simulink model "rfi_blk_v6_casper"  (Simulink 7.1)
settings: Solver=ode45  StartTime=0.0  StopTime=10.0  RelTol=1e-3
undefined symbols (not defined in the file): a, b
libraries: xbsIndex_r4 x13084, xps_library x4
(part 3 of 51)

== system rfi_blk_v6_casper/rfi_blk/mad_comp/Median1/Subsystem11 ==
  data_in : Inport  OutDataType=sfix(16)  OutScaling=2^0
  med_loc : Inport  Port=2  OutDataType=sfix(16)  OutScaling=2^0
  acc_reset : Inport  Port=3  OutDataType=sfix(16)  OutScaling=2^0
  " System Generator" : xbsIndex_r4/ System Generator  Tag=genX  SystemSampleTime=-1  xilinxfamily=Virtex4  part=xc4vsx35  speed=-10  package=ff668  synthesis_tool=XST  clock_wrapper=Clock Enables  directory=./netlist  testbench=off  simulink_period=1  sysclk_period=100  dcm_input_clock_period=100  incr_netlist=off  trim_vbits=Everywhere in SubSystem  dbl_ovrd=According to Block Masks  core_generation=According to Block Masks  run_coregen=off  deprecated_control=off  eval_field=0  block_type=sysgen
      settings: block_version=10.1.3
  Constant10 : xbsIndex_r4/Constant  arith_type=Boolean  const=1  n_bits=1  bin_pt=0  explicit_period=on  period=1  equ=P=C  opselect=C  inp2=PCIN>>17  opr=+  inp1=P  carry=CIN  dbl_ovrd=off  block_type=constant
  Constant11 : xbsIndex_r4/Constant  arith_type=Boolean  const=0  n_bits=1  bin_pt=0  explicit_period=on  period=1  equ=P=C  opselect=C  inp2=PCIN>>17  opr=+  inp1=P  carry=CIN  dbl_ovrd=off  block_type=constant
  Constant12 : xbsIndex_r4/Constant  arith_type=Boolean  const=1  n_bits=1  bin_pt=0  explicit_period=on  period=1  equ=P=C  opselect=C  inp2=PCIN>>17  opr=+  inp1=P  carry=CIN  dbl_ovrd=off  block_type=constant
  Constant2 : xbsIndex_r4/Constant  arith_type=Unsigned  const=0  n_bits=8  bin_pt=0  explicit_period=on  period=1  equ=P=C  opselect=C  inp2=PCIN>>17  opr=+  inp1=P  carry=CIN  dbl_ovrd=off  block_type=constant
  Constant3 : xbsIndex_r4/Constant  arith_type=Unsigned  const=0  n_bits=8  bin_pt=0  explicit_period=on  period=1  equ=P=C  opselect=C  inp2=PCIN>>17  opr=+  inp1=P  carry=CIN  dbl_ovrd=off  block_type=constant
  Constant9 : xbsIndex_r4/Constant  arith_type=Boolean  const=0  n_bits=1  bin_pt=0  explicit_period=on  period=1  equ=P=C  opselect=C  inp2=PCIN>>17  opr=+  inp1=P  carry=CIN  dbl_ovrd=off  block_type=constant
  Inverter : xbsIndex_r4/Inverter  en=off  latency=0  dbl_ovrd=off  block_type=inv
  Logical : xbsIndex_r4/Logical  logical_function=AND  inputs=2  en=off  latency=0  precision=Full  arith_type=Unsigned  n_bits=16  bin_pt=0  align_bp=on  dbl_ovrd=off  block_type=logical
  Logical1 : xbsIndex_r4/Logical  logical_function=OR  inputs=2  en=off  latency=1  precision=User Defined  arith_type=Unsigned  n_bits=1  bin_pt=0  align_bp=on  dbl_ovrd=off  block_type=logical
  Logical2 : xbsIndex_r4/Logical  logical_function=OR  inputs=2  en=off  latency=1  precision=User Defined  arith_type="Signed  (2's comp)"  n_bits=8  bin_pt=0  align_bp=on  dbl_ovrd=off  block_type=logical
  Median_Accumulator1 : Subsystem
  Median_Accumulator2 : Subsystem
  Median_Location1 : Subsystem
  Median_Location2 : Subsystem
  Mux : xbsIndex_r4/Mux  inputs=2  en=off  latency=0  precision=Full  arith_type=Unsigned  n_bits=16  bin_pt=14  quantization=Truncate  overflow=Wrap  dbl_ovrd=off  block_type=mux
  Mux1 : xbsIndex_r4/Mux  inputs=2  en=off  latency=0  precision=Full  arith_type="Signed  (2's comp)"  n_bits=8  bin_pt=0  quantization=Truncate  overflow=Wrap  dbl_ovrd=off  block_type=mux
  Mux2 : xbsIndex_r4/Mux  inputs=2  en=off  latency=0  precision=User Defined  arith_type="Signed  (2's comp)"  n_bits=8  bin_pt=0  quantization=Truncate  overflow=Wrap  dbl_ovrd=off  block_type=mux
  Mux3 : xbsIndex_r4/Mux  inputs=2  en=off  latency=0  precision=User Defined  arith_type="Signed  (2's comp)"  n_bits=8  bin_pt=0  quantization=Truncate  overflow=Wrap  dbl_ovrd=off  block_type=mux
  Scope{1..5} (5 alike) : Scope  NumInputPorts=2
      Scope1 settings: YMin=-5~-5  YMax=5~5
      Scope2 settings: YMin=-5~-5  YMax=5~5
      Scope3 settings: YMin=-5~-5  YMax=5~5  SaveName=ScopeData5
      Scope4 settings: YMin=-5~-5  YMax=5~5  SaveName=ScopeData7
      Scope5 settings: YMin=-5~-5  YMax=5~5  SaveName=ScopeData15
  priority_out1 : Outport  OutDataType=sfix(16)  OutScaling=2^0
  med_calc1 : Outport  Port=2  OutDataType=sfix(16)  OutScaling=2^0
  inc_out : Outport  Port=3
  wires:
  data_in/1 -> Median_Accumulator1/1(data_in), Median_Accumulator2/1(data_in)
  Median_Accumulator1/1(Out1) -> Median_Location1/1(In1), Scope5/1
  Median_Accumulator1/2(Out2) -> Median_Location1/2(In2)
  Median_Accumulator1/3(Out3) -> Median_Location1/3(In3)
  Median_Accumulator1/4(Out4) -> Median_Location1/4(In4)
  Median_Accumulator1/5(Out5) -> Median_Location1/5(In5), Scope5/2
  Median_Accumulator1/6(Out6) -> Median_Location1/6(In6)
  Median_Accumulator1/7(Out7) -> Median_Location1/7(In7)
  Median_Accumulator1/8(Out8) -> Median_Location1/8(In8)
  Median_Location1/1(Out1) -> Scope1/1, Mux2/3
  med_loc/1 -> Median_Location1/9(median_loc), Median_Location2/9(median_loc)
  Median_Accumulator2/8(Out8) -> Median_Location2/8(In8)
  Median_Accumulator2/7(Out7) -> Median_Location2/7(In7)
  Median_Accumulator2/6(Out6) -> Median_Location2/6(In6)
  Median_Accumulator2/5(Out5) -> Median_Location2/5(In5)
  Median_Accumulator2/4(Out4) -> Median_Location2/4(In4)
  Median_Accumulator2/3(Out3) -> Median_Location2/3(In3)
  Median_Accumulator2/2(Out2) -> Median_Location2/2(In2)
  Median_Accumulator2/1(Out1) -> Median_Location2/1(In1)
  Constant9/1 -> Mux/2
  Constant10/1 -> Mux/3
  Mux/1 -> Logical1/1, Mux2/1
  Inverter/1 -> Logical/1
  Logical/1 -> Mux1/1
  Constant11/1 -> Mux1/2
  Mux1/1 -> Logical1/2, Mux3/1
  Median_Location2/1(Out3) -> Mux3/3, Scope2/1, Scope4/2
  Constant12/1 -> Mux1/3
  Mux2/1 -> Logical2/1
  Mux3/1 -> Logical2/2
  Constant2/1 -> Mux2/2
  Constant3/1 -> Mux3/2
  Logical2/1 -> Scope3/2, med_calc1/1
  Logical1/1 -> priority_out1/1, Scope3/1
  Median_Accumulator2/9(inc_signal) -> inc_out/1
  Median_Location1/2(sel1) -> Mux/1, Inverter/1, Scope1/2
  Median_Location2/2(sel2) -> Logical/2, Scope2/2
  acc_reset/1 -> Median_Accumulator1/2(acc_reset), Median_Accumulator2/2(acc_reset)

== system rfi_blk_v6_casper/rfi_blk/mad_comp/Median1/Subsystem12 ==
  data_in : Inport  OutDataType=sfix(16)  OutScaling=2^0
  med_loc : Inport  Port=2  OutDataType=sfix(16)  OutScaling=2^0
  acc_reset : Inport  Port=3  OutDataType=sfix(16)  OutScaling=2^0
  " System Generator" : xbsIndex_r4/ System Generator  Tag=genX  SystemSampleTime=-1  xilinxfamily=Virtex4  part=xc4vsx35  speed=-10  package=ff668  synthesis_tool=XST  clock_wrapper=Clock Enables  directory=./netlist  testbench=off  simulink_period=1  sysclk_period=100  dcm_input_clock_period=100  incr_netlist=off  trim_vbits=Everywhere in SubSystem  dbl_ovrd=According to Block Masks  core_generation=According to Block Masks  run_coregen=off  deprecated_control=off  eval_field=0  block_type=sysgen
      settings: block_version=10.1.3
  Constant10 : xbsIndex_r4/Constant  arith_type=Boolean  const=1  n_bits=1  bin_pt=0  explicit_period=on  period=1  equ=P=C  opselect=C  inp2=PCIN>>17  opr=+  inp1=P  carry=CIN  dbl_ovrd=off  block_type=constant
  Constant11 : xbsIndex_r4/Constant  arith_type=Boolean  const=0  n_bits=1  bin_pt=0  explicit_period=on  period=1  equ=P=C  opselect=C  inp2=PCIN>>17  opr=+  inp1=P  carry=CIN  dbl_ovrd=off  block_type=constant
  Constant12 : xbsIndex_r4/Constant  arith_type=Boolean  const=1  n_bits=1  bin_pt=0  explicit_period=on  period=1  equ=P=C  opselect=C  inp2=PCIN>>17  opr=+  inp1=P  carry=CIN  dbl_ovrd=off  block_type=constant
  Constant2 : xbsIndex_r4/Constant  arith_type=Unsigned  const=0  n_bits=8  bin_pt=0  explicit_period=on  period=1  equ=P=C  opselect=C  inp2=PCIN>>17  opr=+  inp1=P  carry=CIN  dbl_ovrd=off  block_type=constant
  Constant3 : xbsIndex_r4/Constant  arith_type=Unsigned  const=0  n_bits=8  bin_pt=0  explicit_period=on  period=1  equ=P=C  opselect=C  inp2=PCIN>>17  opr=+  inp1=P  carry=CIN  dbl_ovrd=off  block_type=constant
  Constant9 : xbsIndex_r4/Constant  arith_type=Boolean  const=0  n_bits=1  bin_pt=0  explicit_period=on  period=1  equ=P=C  opselect=C  inp2=PCIN>>17  opr=+  inp1=P  carry=CIN  dbl_ovrd=off  block_type=constant
  Inverter : xbsIndex_r4/Inverter  en=off  latency=0  dbl_ovrd=off  block_type=inv
  Logical : xbsIndex_r4/Logical  logical_function=AND  inputs=2  en=off  latency=0  precision=Full  arith_type=Unsigned  n_bits=16  bin_pt=0  align_bp=on  dbl_ovrd=off  block_type=logical
  Logical1 : xbsIndex_r4/Logical  logical_function=OR  inputs=2  en=off  latency=1  precision=User Defined  arith_type=Unsigned  n_bits=1  bin_pt=0  align_bp=on  dbl_ovrd=off  block_type=logical
  Logical2 : xbsIndex_r4/Logical  logical_function=OR  inputs=2  en=off  latency=1  precision=User Defined  arith_type="Signed  (2's comp)"  n_bits=8  bin_pt=0  align_bp=on  dbl_ovrd=off  block_type=logical
  Median_Accumulator1 : Subsystem
  Median_Accumulator2 : Subsystem
  Median_Location1 : Subsystem
  Median_Location2 : Subsystem
  Mux : xbsIndex_r4/Mux  inputs=2  en=off  latency=0  precision=Full  arith_type=Unsigned  n_bits=16  bin_pt=14  quantization=Truncate  overflow=Wrap  dbl_ovrd=off  block_type=mux
  Mux1 : xbsIndex_r4/Mux  inputs=2  en=off  latency=0  precision=Full  arith_type="Signed  (2's comp)"  n_bits=8  bin_pt=0  quantization=Truncate  overflow=Wrap  dbl_ovrd=off  block_type=mux
  Mux2 : xbsIndex_r4/Mux  inputs=2  en=off  latency=0  precision=User Defined  arith_type="Signed  (2's comp)"  n_bits=8  bin_pt=0  quantization=Truncate  overflow=Wrap  dbl_ovrd=off  block_type=mux
  Mux3 : xbsIndex_r4/Mux  inputs=2  en=off  latency=0  precision=User Defined  arith_type="Signed  (2's comp)"  n_bits=8  bin_pt=0  quantization=Truncate  overflow=Wrap  dbl_ovrd=off  block_type=mux
  Scope{1..5} (5 alike) : Scope  NumInputPorts=2
      Scope1 settings: YMin=-5~-5  YMax=5~5
      Scope2 settings: YMin=-5~-5  YMax=5~5
      Scope3 settings: YMin=-5~-5  YMax=5~5  SaveName=ScopeData5
      Scope4 settings: YMin=-5~-5  YMax=5~5  SaveName=ScopeData7
      Scope5 settings: YMin=-5~-5  YMax=5~5  SaveName=ScopeData15
  priority_out1 : Outport  OutDataType=sfix(16)  OutScaling=2^0
  med_calc1 : Outport  Port=2  OutDataType=sfix(16)  OutScaling=2^0
  inc_out : Outport  Port=3
  wires:
  data_in/1 -> Median_Accumulator1/1(data_in), Median_Accumulator2/1(data_in)
  Median_Accumulator1/1(Out1) -> Median_Location1/1(In1), Scope5/1
  Median_Accumulator1/2(Out2) -> Median_Location1/2(In2)
  Median_Accumulator1/3(Out3) -> Median_Location1/3(In3)
  Median_Accumulator1/4(Out4) -> Median_Location1/4(In4)
  Median_Accumulator1/5(Out5) -> Median_Location1/5(In5), Scope5/2
  Median_Accumulator1/6(Out6) -> Median_Location1/6(In6)
  Median_Accumulator1/7(Out7) -> Median_Location1/7(In7)
  Median_Accumulator1/8(Out8) -> Median_Location1/8(In8)
  Median_Location1/1(Out1) -> Scope1/1, Mux2/3
  med_loc/1 -> Median_Location1/9(median_loc), Median_Location2/9(median_loc)
  Median_Accumulator2/8(Out8) -> Median_Location2/8(In8)
  Median_Accumulator2/7(Out7) -> Median_Location2/7(In7)
  Median_Accumulator2/6(Out6) -> Median_Location2/6(In6)
  Median_Accumulator2/5(Out5) -> Median_Location2/5(In5)
  Median_Accumulator2/4(Out4) -> Median_Location2/4(In4)
  Median_Accumulator2/3(Out3) -> Median_Location2/3(In3)
  Median_Accumulator2/2(Out2) -> Median_Location2/2(In2)
  Median_Accumulator2/1(Out1) -> Median_Location2/1(In1)
  Constant9/1 -> Mux/2
  Constant10/1 -> Mux/3
  Mux/1 -> Logical1/1, Mux2/1
  Inverter/1 -> Logical/1
  Logical/1 -> Mux1/1
  Constant11/1 -> Mux1/2
  Mux1/1 -> Logical1/2, Mux3/1
  Median_Location2/1(Out3) -> Mux3/3, Scope2/1, Scope4/2
  Constant12/1 -> Mux1/3
  Mux2/1 -> Logical2/1
  Mux3/1 -> Logical2/2
  Constant2/1 -> Mux2/2
  Constant3/1 -> Mux3/2
  Logical2/1 -> Scope3/2, med_calc1/1
  Logical1/1 -> priority_out1/1, Scope3/1
  Median_Accumulator2/9(inc_signal) -> inc_out/1
  Median_Location1/2(sel1) -> Mux/1, Inverter/1, Scope1/2
  Median_Location2/2(sel2) -> Logical/2, Scope2/2
  acc_reset/1 -> Median_Accumulator1/2(acc_reset), Median_Accumulator2/2(acc_reset)

== system rfi_blk_v6_casper/rfi_blk/mad_comp/Median1/Subsystem13 ==
  data_in : Inport  OutDataType=sfix(16)  OutScaling=2^0
  med_loc : Inport  Port=2  OutDataType=sfix(16)  OutScaling=2^0
  acc_reset : Inport  Port=3  OutDataType=sfix(16)  OutScaling=2^0
  " System Generator" : xbsIndex_r4/ System Generator  Tag=genX  SystemSampleTime=-1  xilinxfamily=Virtex4  part=xc4vsx35  speed=-10  package=ff668  synthesis_tool=XST  clock_wrapper=Clock Enables  directory=./netlist  testbench=off  simulink_period=1  sysclk_period=100  dcm_input_clock_period=100  incr_netlist=off  trim_vbits=Everywhere in SubSystem  dbl_ovrd=According to Block Masks  core_generation=According to Block Masks  run_coregen=off  deprecated_control=off  eval_field=0  block_type=sysgen
      settings: block_version=10.1.3
  Constant10 : xbsIndex_r4/Constant  arith_type=Boolean  const=1  n_bits=1  bin_pt=0  explicit_period=on  period=1  equ=P=C  opselect=C  inp2=PCIN>>17  opr=+  inp1=P  carry=CIN  dbl_ovrd=off  block_type=constant
  Constant11 : xbsIndex_r4/Constant  arith_type=Boolean  const=0  n_bits=1  bin_pt=0  explicit_period=on  period=1  equ=P=C  opselect=C  inp2=PCIN>>17  opr=+  inp1=P  carry=CIN  dbl_ovrd=off  block_type=constant
  Constant12 : xbsIndex_r4/Constant  arith_type=Boolean  const=1  n_bits=1  bin_pt=0  explicit_period=on  period=1  equ=P=C  opselect=C  inp2=PCIN>>17  opr=+  inp1=P  carry=CIN  dbl_ovrd=off  block_type=constant
  Constant2 : xbsIndex_r4/Constant  arith_type=Unsigned  const=0  n_bits=8  bin_pt=0  explicit_period=on  period=1  equ=P=C  opselect=C  inp2=PCIN>>17  opr=+  inp1=P  carry=CIN  dbl_ovrd=off  block_type=constant
  Constant3 : xbsIndex_r4/Constant  arith_type=Unsigned  const=0  n_bits=8  bin_pt=0  explicit_period=on  period=1  equ=P=C  opselect=C  inp2=PCIN>>17  opr=+  inp1=P  carry=CIN  dbl_ovrd=off  block_type=constant
  Constant9 : xbsIndex_r4/Constant  arith_type=Boolean  const=0  n_bits=1  bin_pt=0  explicit_period=on  period=1  equ=P=C  opselect=C  inp2=PCIN>>17  opr=+  inp1=P  carry=CIN  dbl_ovrd=off  block_type=constant
  Inverter : xbsIndex_r4/Inverter  en=off  latency=0  dbl_ovrd=off  block_type=inv
  Logical : xbsIndex_r4/Logical  logical_function=AND  inputs=2  en=off  latency=0  precision=Full  arith_type=Unsigned  n_bits=16  bin_pt=0  align_bp=on  dbl_ovrd=off  block_type=logical
  Logical1 : xbsIndex_r4/Logical  logical_function=OR  inputs=2  en=off  latency=1  precision=User Defined  arith_type=Unsigned  n_bits=1  bin_pt=0  align_bp=on  dbl_ovrd=off  block_type=logical
  Logical2 : xbsIndex_r4/Logical  logical_function=OR  inputs=2  en=off  latency=1  precision=User Defined  arith_type="Signed  (2's comp)"  n_bits=8  bin_pt=0  align_bp=on  dbl_ovrd=off  block_type=logical
  Median_Accumulator1 : Subsystem
  Median_Accumulator2 : Subsystem
  Median_Location1 : Subsystem
  Median_Location2 : Subsystem
  Mux : xbsIndex_r4/Mux  inputs=2  en=off  latency=0  precision=Full  arith_type=Unsigned  n_bits=16  bin_pt=14  quantization=Truncate  overflow=Wrap  dbl_ovrd=off  block_type=mux
  Mux1 : xbsIndex_r4/Mux  inputs=2  en=off  latency=0  precision=Full  arith_type="Signed  (2's comp)"  n_bits=8  bin_pt=0  quantization=Truncate  overflow=Wrap  dbl_ovrd=off  block_type=mux
  Mux2 : xbsIndex_r4/Mux  inputs=2  en=off  latency=0  precision=User Defined  arith_type="Signed  (2's comp)"  n_bits=8  bin_pt=0  quantization=Truncate  overflow=Wrap  dbl_ovrd=off  block_type=mux
  Mux3 : xbsIndex_r4/Mux  inputs=2  en=off  latency=0  precision=User Defined  arith_type="Signed  (2's comp)"  n_bits=8  bin_pt=0  quantization=Truncate  overflow=Wrap  dbl_ovrd=off  block_type=mux
  Scope{1..5} (5 alike) : Scope  NumInputPorts=2
      Scope1 settings: YMin=-5~-5  YMax=5~5
      Scope2 settings: YMin=-5~-5  YMax=5~5
      Scope3 settings: YMin=-5~-5  YMax=5~5  SaveName=ScopeData5
      Scope4 settings: YMin=-5~-5  YMax=5~5  SaveName=ScopeData7
      Scope5 settings: YMin=-5~-5  YMax=5~5  SaveName=ScopeData15
  priority_out1 : Outport  OutDataType=sfix(16)  OutScaling=2^0
  med_calc1 : Outport  Port=2  OutDataType=sfix(16)  OutScaling=2^0
  inc_out : Outport  Port=3
  wires:
  data_in/1 -> Median_Accumulator1/1(data_in), Median_Accumulator2/1(data_in)
  Median_Accumulator1/1(Out1) -> Median_Location1/1(In1), Scope5/1
  Median_Accumulator1/2(Out2) -> Median_Location1/2(In2)
  Median_Accumulator1/3(Out3) -> Median_Location1/3(In3)
  Median_Accumulator1/4(Out4) -> Median_Location1/4(In4)
  Median_Accumulator1/5(Out5) -> Median_Location1/5(In5), Scope5/2
  Median_Accumulator1/6(Out6) -> Median_Location1/6(In6)
  Median_Accumulator1/7(Out7) -> Median_Location1/7(In7)
  Median_Accumulator1/8(Out8) -> Median_Location1/8(In8)
  Median_Location1/1(Out1) -> Scope1/1, Mux2/3
  med_loc/1 -> Median_Location1/9(median_loc), Median_Location2/9(median_loc)
  Median_Accumulator2/8(Out8) -> Median_Location2/8(In8)
  Median_Accumulator2/7(Out7) -> Median_Location2/7(In7)
  Median_Accumulator2/6(Out6) -> Median_Location2/6(In6)
  Median_Accumulator2/5(Out5) -> Median_Location2/5(In5)
  Median_Accumulator2/4(Out4) -> Median_Location2/4(In4)
  Median_Accumulator2/3(Out3) -> Median_Location2/3(In3)
  Median_Accumulator2/2(Out2) -> Median_Location2/2(In2)
  Median_Accumulator2/1(Out1) -> Median_Location2/1(In1)
  Constant9/1 -> Mux/2
  Constant10/1 -> Mux/3
  Mux/1 -> Logical1/1, Mux2/1
  Inverter/1 -> Logical/1
  Logical/1 -> Mux1/1
  Constant11/1 -> Mux1/2
  Mux1/1 -> Logical1/2, Mux3/1
  Median_Location2/1(Out3) -> Mux3/3, Scope2/1, Scope4/2
  Constant12/1 -> Mux1/3
  Mux2/1 -> Logical2/1
  Mux3/1 -> Logical2/2
  Constant2/1 -> Mux2/2
  Constant3/1 -> Mux3/2
  Logical2/1 -> Scope3/2, med_calc1/1
  Logical1/1 -> priority_out1/1, Scope3/1
  Median_Accumulator2/9(inc_signal) -> inc_out/1
  Median_Location1/2(sel1) -> Mux/1, Inverter/1, Scope1/2
  Median_Location2/2(sel2) -> Logical/2, Scope2/2
  acc_reset/1 -> Median_Accumulator1/2(acc_reset), Median_Accumulator2/2(acc_reset)

== system rfi_blk_v6_casper/rfi_blk/mad_comp/Median1/Subsystem14 ==
  data_in : Inport  OutDataType=sfix(16)  OutScaling=2^0
  med_loc : Inport  Port=2  OutDataType=sfix(16)  OutScaling=2^0
  acc_reset : Inport  Port=3  OutDataType=sfix(16)  OutScaling=2^0
  " System Generator" : xbsIndex_r4/ System Generator  Tag=genX  SystemSampleTime=-1  xilinxfamily=Virtex4  part=xc4vsx35  speed=-10  package=ff668  synthesis_tool=XST  clock_wrapper=Clock Enables  directory=./netlist  testbench=off  simulink_period=1  sysclk_period=100  dcm_input_clock_period=100  incr_netlist=off  trim_vbits=Everywhere in SubSystem  dbl_ovrd=According to Block Masks  core_generation=According to Block Masks  run_coregen=off  deprecated_control=off  eval_field=0  block_type=sysgen
      settings: block_version=10.1.3
  Constant10 : xbsIndex_r4/Constant  arith_type=Boolean  const=1  n_bits=1  bin_pt=0  explicit_period=on  period=1  equ=P=C  opselect=C  inp2=PCIN>>17  opr=+  inp1=P  carry=CIN  dbl_ovrd=off  block_type=constant
  Constant11 : xbsIndex_r4/Constant  arith_type=Boolean  const=0  n_bits=1  bin_pt=0  explicit_period=on  period=1  equ=P=C  opselect=C  inp2=PCIN>>17  opr=+  inp1=P  carry=CIN  dbl_ovrd=off  block_type=constant
  Constant12 : xbsIndex_r4/Constant  arith_type=Boolean  const=1  n_bits=1  bin_pt=0  explicit_period=on  period=1  equ=P=C  opselect=C  inp2=PCIN>>17  opr=+  inp1=P  carry=CIN  dbl_ovrd=off  block_type=constant
  Constant2 : xbsIndex_r4/Constant  arith_type=Unsigned  const=0  n_bits=8  bin_pt=0  explicit_period=on  period=1  equ=P=C  opselect=C  inp2=PCIN>>17  opr=+  inp1=P  carry=CIN  dbl_ovrd=off  block_type=constant
  Constant3 : xbsIndex_r4/Constant  arith_type=Unsigned  const=0  n_bits=8  bin_pt=0  explicit_period=on  period=1  equ=P=C  opselect=C  inp2=PCIN>>17  opr=+  inp1=P  carry=CIN  dbl_ovrd=off  block_type=constant
  Constant9 : xbsIndex_r4/Constant  arith_type=Boolean  const=0  n_bits=1  bin_pt=0  explicit_period=on  period=1  equ=P=C  opselect=C  inp2=PCIN>>17  opr=+  inp1=P  carry=CIN  dbl_ovrd=off  block_type=constant
  Inverter : xbsIndex_r4/Inverter  en=off  latency=0  dbl_ovrd=off  block_type=inv
  Logical : xbsIndex_r4/Logical  logical_function=AND  inputs=2  en=off  latency=0  precision=Full  arith_type=Unsigned  n_bits=16  bin_pt=0  align_bp=on  dbl_ovrd=off  block_type=logical
  Logical1 : xbsIndex_r4/Logical  logical_function=OR  inputs=2  en=off  latency=1  precision=User Defined  arith_type=Unsigned  n_bits=1  bin_pt=0  align_bp=on  dbl_ovrd=off  block_type=logical
  Logical2 : xbsIndex_r4/Logical  logical_function=OR  inputs=2  en=off  latency=1  precision=User Defined  arith_type="Signed  (2's comp)"  n_bits=8  bin_pt=0  align_bp=on  dbl_ovrd=off  block_type=logical
  Median_Accumulator1 : Subsystem
  Median_Accumulator2 : Subsystem
  Median_Location1 : Subsystem
  Median_Location2 : Subsystem
  Mux : xbsIndex_r4/Mux  inputs=2  en=off  latency=0  precision=Full  arith_type=Unsigned  n_bits=16  bin_pt=14  quantization=Truncate  overflow=Wrap  dbl_ovrd=off  block_type=mux
  Mux1 : xbsIndex_r4/Mux  inputs=2  en=off  latency=0  precision=Full  arith_type="Signed  (2's comp)"  n_bits=8  bin_pt=0  quantization=Truncate  overflow=Wrap  dbl_ovrd=off  block_type=mux
  Mux2 : xbsIndex_r4/Mux  inputs=2  en=off  latency=0  precision=User Defined  arith_type="Signed  (2's comp)"  n_bits=8  bin_pt=0  quantization=Truncate  overflow=Wrap  dbl_ovrd=off  block_type=mux
  Mux3 : xbsIndex_r4/Mux  inputs=2  en=off  latency=0  precision=User Defined  arith_type="Signed  (2's comp)"  n_bits=8  bin_pt=0  quantization=Truncate  overflow=Wrap  dbl_ovrd=off  block_type=mux
  Scope{1..5} (5 alike) : Scope  NumInputPorts=2
      Scope1 settings: YMin=-5~-5  YMax=5~5
      Scope2 settings: YMin=-5~-5  YMax=5~5
      Scope3 settings: YMin=-5~-5  YMax=5~5  SaveName=ScopeData5
      Scope4 settings: YMin=-5~-5  YMax=5~5  SaveName=ScopeData7
      Scope5 settings: YMin=-5~-5  YMax=5~5  SaveName=ScopeData15
  priority_out1 : Outport  OutDataType=sfix(16)  OutScaling=2^0
  med_calc1 : Outport  Port=2  OutDataType=sfix(16)  OutScaling=2^0
  inc_out : Outport  Port=3
  wires:
  data_in/1 -> Median_Accumulator1/1(data_in), Median_Accumulator2/1(data_in)
  Median_Accumulator1/1(Out1) -> Median_Location1/1(In1), Scope5/1
  Median_Accumulator1/2(Out2) -> Median_Location1/2(In2)
  Median_Accumulator1/3(Out3) -> Median_Location1/3(In3)
  Median_Accumulator1/4(Out4) -> Median_Location1/4(In4)
  Median_Accumulator1/5(Out5) -> Median_Location1/5(In5), Scope5/2
  Median_Accumulator1/6(Out6) -> Median_Location1/6(In6)
  Median_Accumulator1/7(Out7) -> Median_Location1/7(In7)
  Median_Accumulator1/8(Out8) -> Median_Location1/8(In8)
  Median_Location1/1(Out1) -> Scope1/1, Mux2/3
  med_loc/1 -> Median_Location1/9(median_loc), Median_Location2/9(median_loc)
  Median_Accumulator2/8(Out8) -> Median_Location2/8(In8)
  Median_Accumulator2/7(Out7) -> Median_Location2/7(In7)
  Median_Accumulator2/6(Out6) -> Median_Location2/6(In6)
  Median_Accumulator2/5(Out5) -> Median_Location2/5(In5)
  Median_Accumulator2/4(Out4) -> Median_Location2/4(In4)
  Median_Accumulator2/3(Out3) -> Median_Location2/3(In3)
  Median_Accumulator2/2(Out2) -> Median_Location2/2(In2)
  Median_Accumulator2/1(Out1) -> Median_Location2/1(In1)
  Constant9/1 -> Mux/2
  Constant10/1 -> Mux/3
  Mux/1 -> Logical1/1, Mux2/1
  Inverter/1 -> Logical/1
  Logical/1 -> Mux1/1
  Constant11/1 -> Mux1/2
  Mux1/1 -> Logical1/2, Mux3/1
  Median_Location2/1(Out3) -> Mux3/3, Scope2/1, Scope4/2
  Constant12/1 -> Mux1/3
  Mux2/1 -> Logical2/1
  Mux3/1 -> Logical2/2
  Constant2/1 -> Mux2/2
  Constant3/1 -> Mux3/2
  Logical2/1 -> Scope3/2, med_calc1/1
  Logical1/1 -> priority_out1/1, Scope3/1
  Median_Accumulator2/9(inc_signal) -> inc_out/1
  Median_Location1/2(sel1) -> Mux/1, Inverter/1, Scope1/2
  Median_Location2/2(sel2) -> Logical/2, Scope2/2
  acc_reset/1 -> Median_Accumulator1/2(acc_reset), Median_Accumulator2/2(acc_reset)

== system rfi_blk_v6_casper/rfi_blk/mad_comp/Median1/Subsystem15 ==
  data_in : Inport  OutDataType=sfix(16)  OutScaling=2^0
  med_loc : Inport  Port=2  OutDataType=sfix(16)  OutScaling=2^0
  acc_reset : Inport  Port=3  OutDataType=sfix(16)  OutScaling=2^0
  " System Generator" : xbsIndex_r4/ System Generator  Tag=genX  SystemSampleTime=-1  xilinxfamily=Virtex4  part=xc4vsx35  speed=-10  package=ff668  synthesis_tool=XST  clock_wrapper=Clock Enables  directory=./netlist  testbench=off  simulink_period=1  sysclk_period=100  dcm_input_clock_period=100  incr_netlist=off  trim_vbits=Everywhere in SubSystem  dbl_ovrd=According to Block Masks  core_generation=According to Block Masks  run_coregen=off  deprecated_control=off  eval_field=0  block_type=sysgen
      settings: block_version=10.1.3
  Constant10 : xbsIndex_r4/Constant  arith_type=Boolean  const=1  n_bits=1  bin_pt=0  explicit_period=on  period=1  equ=P=C  opselect=C  inp2=PCIN>>17  opr=+  inp1=P  carry=CIN  dbl_ovrd=off  block_type=constant
  Constant11 : xbsIndex_r4/Constant  arith_type=Boolean  const=0  n_bits=1  bin_pt=0  explicit_period=on  period=1  equ=P=C  opselect=C  inp2=PCIN>>17  opr=+  inp1=P  carry=CIN  dbl_ovrd=off  block_type=constant
  Constant12 : xbsIndex_r4/Constant  arith_type=Boolean  const=1  n_bits=1  bin_pt=0  explicit_period=on  period=1  equ=P=C  opselect=C  inp2=PCIN>>17  opr=+  inp1=P  carry=CIN  dbl_ovrd=off  block_type=constant
  Constant2 : xbsIndex_r4/Constant  arith_type=Unsigned  const=0  n_bits=8  bin_pt=0  explicit_period=on  period=1  equ=P=C  opselect=C  inp2=PCIN>>17  opr=+  inp1=P  carry=CIN  dbl_ovrd=off  block_type=constant
  Constant3 : xbsIndex_r4/Constant  arith_type=Unsigned  const=0  n_bits=8  bin_pt=0  explicit_period=on  period=1  equ=P=C  opselect=C  inp2=PCIN>>17  opr=+  inp1=P  carry=CIN  dbl_ovrd=off  block_type=constant
  Constant9 : xbsIndex_r4/Constant  arith_type=Boolean  const=0  n_bits=1  bin_pt=0  explicit_period=on  period=1  equ=P=C  opselect=C  inp2=PCIN>>17  opr=+  inp1=P  carry=CIN  dbl_ovrd=off  block_type=constant
  Inverter : xbsIndex_r4/Inverter  en=off  latency=0  dbl_ovrd=off  block_type=inv
  Logical : xbsIndex_r4/Logical  logical_function=AND  inputs=2  en=off  latency=0  precision=Full  arith_type=Unsigned  n_bits=16  bin_pt=0  align_bp=on  dbl_ovrd=off  block_type=logical
  Logical1 : xbsIndex_r4/Logical  logical_function=OR  inputs=2  en=off  latency=1  precision=User Defined  arith_type=Unsigned  n_bits=1  bin_pt=0  align_bp=on  dbl_ovrd=off  block_type=logical
  Logical2 : xbsIndex_r4/Logical  logical_function=OR  inputs=2  en=off  latency=1  precision=User Defined  arith_type="Signed  (2's comp)"  n_bits=8  bin_pt=0  align_bp=on  dbl_ovrd=off  block_type=logical
  Median_Accumulator1 : Subsystem
  Median_Accumulator2 : Subsystem
  Median_Location1 : Subsystem
  Median_Location2 : Subsystem
  Mux : xbsIndex_r4/Mux  inputs=2  en=off  latency=0  precision=Full  arith_type=Unsigned  n_bits=16  bin_pt=14  quantization=Truncate  overflow=Wrap  dbl_ovrd=off  block_type=mux
  Mux1 : xbsIndex_r4/Mux  inputs=2  en=off  latency=0  precision=Full  arith_type="Signed  (2's comp)"  n_bits=8  bin_pt=0  quantization=Truncate  overflow=Wrap  dbl_ovrd=off  block_type=mux
  Mux2 : xbsIndex_r4/Mux  inputs=2  en=off  latency=0  precision=User Defined  arith_type="Signed  (2's comp)"  n_bits=8  bin_pt=0  quantization=Truncate  overflow=Wrap  dbl_ovrd=off  block_type=mux
  Mux3 : xbsIndex_r4/Mux  inputs=2  en=off  latency=0  precision=User Defined  arith_type="Signed  (2's comp)"  n_bits=8  bin_pt=0  quantization=Truncate  overflow=Wrap  dbl_ovrd=off  block_type=mux
  Scope{1..5} (5 alike) : Scope  NumInputPorts=2
      Scope1 settings: YMin=-5~-5  YMax=5~5
      Scope2 settings: YMin=-5~-5  YMax=5~5
      Scope3 settings: YMin=-5~-5  YMax=5~5  SaveName=ScopeData5
      Scope4 settings: YMin=-5~-5  YMax=5~5  SaveName=ScopeData7
      Scope5 settings: YMin=-5~-5  YMax=5~5  SaveName=ScopeData15
  priority_out1 : Outport  OutDataType=sfix(16)  OutScaling=2^0
  med_calc1 : Outport  Port=2  OutDataType=sfix(16)  OutScaling=2^0
  inc_out : Outport  Port=3
  wires:
  data_in/1 -> Median_Accumulator1/1(data_in), Median_Accumulator2/1(data_in)
  Median_Accumulator1/1(Out1) -> Median_Location1/1(In1), Scope5/1
  Median_Accumulator1/2(Out2) -> Median_Location1/2(In2)
  Median_Accumulator1/3(Out3) -> Median_Location1/3(In3)
  Median_Accumulator1/4(Out4) -> Median_Location1/4(In4)
  Median_Accumulator1/5(Out5) -> Median_Location1/5(In5), Scope5/2
  Median_Accumulator1/6(Out6) -> Median_Location1/6(In6)
  Median_Accumulator1/7(Out7) -> Median_Location1/7(In7)
  Median_Accumulator1/8(Out8) -> Median_Location1/8(In8)
  Median_Location1/1(Out1) -> Scope1/1, Mux2/3
  med_loc/1 -> Median_Location1/9(median_loc), Median_Location2/9(median_loc)
  Median_Accumulator2/8(Out8) -> Median_Location2/8(In8)
  Median_Accumulator2/7(Out7) -> Median_Location2/7(In7)
  Median_Accumulator2/6(Out6) -> Median_Location2/6(In6)
  Median_Accumulator2/5(Out5) -> Median_Location2/5(In5)
  Median_Accumulator2/4(Out4) -> Median_Location2/4(In4)
  Median_Accumulator2/3(Out3) -> Median_Location2/3(In3)
  Median_Accumulator2/2(Out2) -> Median_Location2/2(In2)
  Median_Accumulator2/1(Out1) -> Median_Location2/1(In1)
  Constant9/1 -> Mux/2
  Constant10/1 -> Mux/3
  Mux/1 -> Logical1/1, Mux2/1
  Inverter/1 -> Logical/1
  Logical/1 -> Mux1/1
  Constant11/1 -> Mux1/2
  Mux1/1 -> Logical1/2, Mux3/1
  Median_Location2/1(Out3) -> Mux3/3, Scope2/1, Scope4/2
  Constant12/1 -> Mux1/3
  Mux2/1 -> Logical2/1
  Mux3/1 -> Logical2/2
  Constant2/1 -> Mux2/2
  Constant3/1 -> Mux3/2
  Logical2/1 -> Scope3/2, med_calc1/1
  Logical1/1 -> priority_out1/1, Scope3/1
  Median_Accumulator2/9(inc_signal) -> inc_out/1
  Median_Location1/2(sel1) -> Mux/1, Inverter/1, Scope1/2
  Median_Location2/2(sel2) -> Logical/2, Scope2/2
  acc_reset/1 -> Median_Accumulator1/2(acc_reset), Median_Accumulator2/2(acc_reset)

== system rfi_blk_v6_casper/rfi_blk/mad_comp/Median1/Subsystem16 ==
  med_sel1 : Inport
  med_sel2 : Inport  Port=2
  med_sel3 : Inport  Port=3
  med_sel4 : Inport  Port=4
  med_sel5 : Inport  Port=5
  med_sel6 : Inport  Port=6
  med_sel7 : Inport  Port=7
  med_sel8 : Inport  Port=8
  med_in1 : Inport  Port=9
  med_in2 : Inport  Port=10
  med_in3 : Inport  Port=11
  med_in4 : Inport  Port=12
  med_in5 : Inport  Port=13
  med_in6 : Inport  Port=14
  med_in7 : Inport  Port=15
  med_in8 : Inport  Port=16
  med_sel9 : Inport  Port=17
  med_sel10 : Inport  Port=18
  med_sel11 : Inport  Port=19
  med_sel12 : Inport  Port=20
  med_sel13 : Inport  Port=21
  med_sel14 : Inport  Port=22
  med_sel15 : Inport  Port=23
  med_sel16 : Inport  Port=24
  med_in9 : Inport  Port=25
  med_in10 : Inport  Port=26
  med_in11 : Inport  Port=27
  med_in12 : Inport  Port=28
  med_in13 : Inport  Port=29
  med_in14 : Inport  Port=30
  med_in15 : Inport  Port=31
  med_in16 : Inport  Port=32
  Constant10 : xbsIndex_r4/Constant  arith_type=Boolean  const=1  n_bits=1  bin_pt=0  explicit_period=on  period=1  equ=P=C  opselect=C  inp2=PCIN>>17  opr=+  inp1=P  carry=CIN  dbl_ovrd=off  block_type=constant
  Constant11 : xbsIndex_r4/Constant  arith_type=Boolean  const=0  n_bits=1  bin_pt=0  explicit_period=on  period=1  equ=P=C  opselect=C  inp2=PCIN>>17  opr=+  inp1=P  carry=CIN  dbl_ovrd=off  block_type=constant
  Constant12 : xbsIndex_r4/Constant  arith_type=Boolean  const=1  n_bits=1  bin_pt=0  explicit_period=on  period=1  equ=P=C  opselect=C  inp2=PCIN>>17  opr=+  inp1=P  carry=CIN  dbl_ovrd=off  block_type=constant
  Constant2 : xbsIndex_r4/Constant  arith_type=Unsigned  const=0  n_bits=8  bin_pt=0  explicit_period=on  period=1  equ=P=C  opselect=C  inp2=PCIN>>17  opr=+  inp1=P  carry=CIN  dbl_ovrd=off  block_type=constant
  Constant3 : xbsIndex_r4/Constant  arith_type=Unsigned  const=0  n_bits=8  bin_pt=0  explicit_period=on  period=1  equ=P=C  opselect=C  inp2=PCIN>>17  opr=+  inp1=P  carry=CIN  dbl_ovrd=off  block_type=constant
  Constant9 : xbsIndex_r4/Constant  arith_type=Boolean  const=0  n_bits=1  bin_pt=0  explicit_period=on  period=1  equ=P=C  opselect=C  inp2=PCIN>>17  opr=+  inp1=P  carry=CIN  dbl_ovrd=off  block_type=constant
  Inverter : xbsIndex_r4/Inverter  en=off  latency=0  dbl_ovrd=off  block_type=inv
  Logical : xbsIndex_r4/Logical  logical_function=AND  inputs=2  en=off  latency=0  precision=Full  arith_type=Unsigned  n_bits=16  bin_pt=0  align_bp=on  dbl_ovrd=off  block_type=logical
  Logical1 : xbsIndex_r4/Logical  logical_function=OR  inputs=2  en=off  latency=1  precision=User Defined  arith_type=Unsigned  n_bits=1  bin_pt=0  align_bp=on  dbl_ovrd=off  block_type=logical
  Logical2 : xbsIndex_r4/Logical  logical_function=OR  inputs=2  en=off  latency=1  precision=User Defined  arith_type="Signed  (2's comp)"  n_bits=8  bin_pt=0  align_bp=on  dbl_ovrd=off  block_type=logical
  Median_Location1 : Subsystem
  Median_Location2 : Subsystem
  Mux : xbsIndex_r4/Mux  inputs=2  en=off  latency=0  precision=Full  arith_type=Unsigned  n_bits=16  bin_pt=14  quantization=Truncate  overflow=Wrap  dbl_ovrd=off  block_type=mux
  Mux1 : xbsIndex_r4/Mux  inputs=2  en=off  latency=0  precision=Full  arith_type=Unsigned  n_bits=16  bin_pt=14  quantization=Truncate  overflow=Wrap  dbl_ovrd=off  block_type=mux
  Mux2 : xbsIndex_r4/Mux  inputs=2  en=off  latency=0  precision=User Defined  arith_type="Signed  (2's comp)"  n_bits=8  bin_pt=0  quantization=Truncate  overflow=Wrap  dbl_ovrd=off  block_type=mux
  Mux3 : xbsIndex_r4/Mux  inputs=2  en=off  latency=0  precision=User Defined  arith_type="Signed  (2's comp)"  n_bits=8  bin_pt=0  quantization=Truncate  overflow=Wrap  dbl_ovrd=off  block_type=mux
  Scope : Scope  NumInputPorts=3
      settings: YMin=-5~-5~-5  YMax=5~5~5  SaveName=ScopeData8
  Scope1 : Scope
      settings: SaveName=ScopeData10
  Scope2 : Scope  NumInputPorts=16
      settings: YMin=-5~-5~-5~-5~-5~-5~-5~-5~-5~-5~-5~-5~-5~-5~-5~-5  YMax=5~5~5~5~5~5~5~5~5~5~5~5~5~5~5~5  SaveName=ScopeData11
  Scope3 : Scope  NumInputPorts=2
      settings: YMin=-5~-5  YMax=5~5
  Scope4 : Scope
      settings: SaveName=ScopeData12
  median_out : Outport  OutDataType=sfix(16)  OutScaling=2^0
  median_out_valid : Outport  Port=2  OutDataType=sfix(16)  OutScaling=2^0
  wires:
  Median_Location1/1(median_final1) -> Mux2/3, Scope/2
  Constant9/1 -> Mux/2
  Constant10/1 -> Mux/3
  Mux/1 -> Logical1/1, Mux2/1
  Inverter/1 -> Logical/1
  Logical/1 -> Mux1/1
  Constant11/1 -> Mux1/2
  Mux1/1 -> Logical1/2, Mux3/1
  Median_Location2/1(median_final2) -> Mux3/3, Scope/3
  Constant12/1 -> Mux1/3
  Mux2/1 -> Logical2/1
  Mux3/1 -> Logical2/2
  Constant2/1 -> Mux2/2
  Constant3/1 -> Mux3/2
  Logical2/1 -> Scope3/2, median_out/1, Scope/1
  Logical1/1 -> Scope3/1, median_out_valid/1
  Median_Location1/2(median_valid1) -> Mux/1, Inverter/1
  Median_Location2/2(median_valid2) -> Logical/2
  med_sel1/1 -> Median_Location1/1(med_sel1), Scope2/1
  med_sel2/1 -> Median_Location1/2(med_sel2), Scope2/2
  med_sel3/1 -> Median_Location1/3(med_sel3), Scope2/3
  med_sel4/1 -> Median_Location1/4(med_sel4), Scope2/4
  med_sel5/1 -> Median_Location1/5(med_sel5), Scope2/5
  med_sel6/1 -> Median_Location1/6(med_sel6), Scope2/6
  med_sel7/1 -> Median_Location1/7(med_sel7), Scope2/7
  med_in1/1 -> Median_Location1/9(med_in1), Scope2/9
  med_in2/1 -> Median_Location1/10(med_in2)
  med_in3/1 -> Median_Location1/11(med_in3)
  med_in4/1 -> Median_Location1/12(med_in4)
  med_in5/1 -> Median_Location1/13(med_in5)
  med_in6/1 -> Median_Location1/14(med_in6)
  med_in7/1 -> Median_Location1/15(med_in7)
  med_in8/1 -> Median_Location1/16(med_in8)
  med_sel9/1 -> Median_Location2/1(med_sel9)
  med_sel10/1 -> Median_Location2/2(med_sel10)
  med_sel11/1 -> Median_Location2/3(med_sel11)
  med_sel12/1 -> Median_Location2/4(med_sel12)
  med_sel13/1 -> Median_Location2/5(med_sel13)
  med_sel14/1 -> Median_Location2/6(med_sel14)
  med_sel15/1 -> Median_Location2/7(med_sel15)
  med_sel16/1 -> Median_Location2/8(med_sel16)
  med_in9/1 -> Median_Location2/9(med_in9)
  med_in10/1 -> Median_Location2/10(med_in10)
  med_in11/1 -> Median_Location2/11(med_in11)
  med_in12/1 -> Median_Location2/12(med_in12)
  med_in13/1 -> Median_Location2/13(med_in13)
  med_in14/1 -> Median_Location2/14(med_in14)
  med_in15/1 -> Median_Location2/15(med_in15)
  med_in16/1 -> Median_Location2/16(med_in16)
  med_sel8/1 -> Median_Location1/8(med_sel8), Scope2/8, Scope4/1

== system rfi_blk_v6_casper/rfi_blk/mad_comp/Median1/Subsystem2 ==
  data_in : Inport  OutDataType=sfix(16)  OutScaling=2^0
  med_loc : Inport  Port=2  OutDataType=sfix(16)  OutScaling=2^0
  acc_reset : Inport  Port=3  OutDataType=sfix(16)  OutScaling=2^0
  " System Generator" : xbsIndex_r4/ System Generator  Tag=genX  SystemSampleTime=-1  xilinxfamily=Virtex4  part=xc4vsx35  speed=-10  package=ff668  synthesis_tool=XST  clock_wrapper=Clock Enables  directory=./netlist  testbench=off  simulink_period=1  sysclk_period=100  dcm_input_clock_period=100  incr_netlist=off  trim_vbits=Everywhere in SubSystem  dbl_ovrd=According to Block Masks  core_generation=According to Block Masks  run_coregen=off  deprecated_control=off  eval_field=0  block_type=sysgen
      settings: block_version=10.1.3
  Constant10 : xbsIndex_r4/Constant  arith_type=Boolean  const=1  n_bits=1  bin_pt=0  explicit_period=on  period=1  equ=P=C  opselect=C  inp2=PCIN>>17  opr=+  inp1=P  carry=CIN  dbl_ovrd=off  block_type=constant
  Constant11 : xbsIndex_r4/Constant  arith_type=Boolean  const=0  n_bits=1  bin_pt=0  explicit_period=on  period=1  equ=P=C  opselect=C  inp2=PCIN>>17  opr=+  inp1=P  carry=CIN  dbl_ovrd=off  block_type=constant
  Constant12 : xbsIndex_r4/Constant  arith_type=Boolean  const=1  n_bits=1  bin_pt=0  explicit_period=on  period=1  equ=P=C  opselect=C  inp2=PCIN>>17  opr=+  inp1=P  carry=CIN  dbl_ovrd=off  block_type=constant
  Constant2 : xbsIndex_r4/Constant  arith_type=Unsigned  const=0  n_bits=8  bin_pt=0  explicit_period=on  period=1  equ=P=C  opselect=C  inp2=PCIN>>17  opr=+  inp1=P  carry=CIN  dbl_ovrd=off  block_type=constant
  Constant3 : xbsIndex_r4/Constant  arith_type=Unsigned  const=0  n_bits=8  bin_pt=0  explicit_period=on  period=1  equ=P=C  opselect=C  inp2=PCIN>>17  opr=+  inp1=P  carry=CIN  dbl_ovrd=off  block_type=constant
  Constant9 : xbsIndex_r4/Constant  arith_type=Boolean  const=0  n_bits=1  bin_pt=0  explicit_period=on  period=1  equ=P=C  opselect=C  inp2=PCIN>>17  opr=+  inp1=P  carry=CIN  dbl_ovrd=off  block_type=constant
  Inverter : xbsIndex_r4/Inverter  en=off  latency=0  dbl_ovrd=off  block_type=inv
  Logical : xbsIndex_r4/Logical  logical_function=AND  inputs=2  en=off  latency=0  precision=Full  arith_type=Unsigned  n_bits=16  bin_pt=0  align_bp=on  dbl_ovrd=off  block_type=logical
  Logical1 : xbsIndex_r4/Logical  logical_function=OR  inputs=2  en=off  latency=1  precision=User Defined  arith_type=Unsigned  n_bits=1  bin_pt=0  align_bp=on  dbl_ovrd=off  block_type=logical
  Logical2 : xbsIndex_r4/Logical  logical_function=OR  inputs=2  en=off  latency=1  precision=User Defined  arith_type="Signed  (2's comp)"  n_bits=8  bin_pt=0  align_bp=on  dbl_ovrd=off  block_type=logical
  Median_Accumulator1 : Subsystem
  Median_Accumulator2 : Subsystem
  Median_Location1 : Subsystem
  Median_Location2 : Subsystem
  Mux : xbsIndex_r4/Mux  inputs=2  en=off  latency=0  precision=Full  arith_type=Unsigned  n_bits=16  bin_pt=14  quantization=Truncate  overflow=Wrap  dbl_ovrd=off  block_type=mux
  Mux1 : xbsIndex_r4/Mux  inputs=2  en=off  latency=0  precision=Full  arith_type="Signed  (2's comp)"  n_bits=8  bin_pt=0  quantization=Truncate  overflow=Wrap  dbl_ovrd=off  block_type=mux
  Mux2 : xbsIndex_r4/Mux  inputs=2  en=off  latency=0  precision=User Defined  arith_type="Signed  (2's comp)"  n_bits=8  bin_pt=0  quantization=Truncate  overflow=Wrap  dbl_ovrd=off  block_type=mux
  Mux3 : xbsIndex_r4/Mux  inputs=2  en=off  latency=0  precision=User Defined  arith_type="Signed  (2's comp)"  n_bits=8  bin_pt=0  quantization=Truncate  overflow=Wrap  dbl_ovrd=off  block_type=mux
  Scope{1..5} (5 alike) : Scope  NumInputPorts=2
      Scope1 settings: YMin=-5~-5  YMax=5~5
      Scope2 settings: YMin=-5~-5  YMax=5~5
      Scope3 settings: YMin=-5~-5  YMax=5~5  SaveName=ScopeData5
      Scope4 settings: YMin=-5~-5  YMax=5~5  SaveName=ScopeData7
      Scope5 settings: YMin=-5~-5  YMax=5~5  SaveName=ScopeData15
  priority_out1 : Outport  OutDataType=sfix(16)  OutScaling=2^0
  med_calc1 : Outport  Port=2  OutDataType=sfix(16)  OutScaling=2^0
  inc_out : Outport  Port=3
  wires:
  data_in/1 -> Median_Accumulator1/1(data_in), Median_Accumulator2/1(data_in)
  Median_Accumulator1/1(Out1) -> Median_Location1/1(In1), Scope5/1
  Median_Accumulator1/2(Out2) -> Median_Location1/2(In2)
  Median_Accumulator1/3(Out3) -> Median_Location1/3(In3)
  Median_Accumulator1/4(Out4) -> Median_Location1/4(In4)
  Median_Accumulator1/5(Out5) -> Median_Location1/5(In5), Scope5/2
  Median_Accumulator1/6(Out6) -> Median_Location1/6(In6)
  Median_Accumulator1/7(Out7) -> Median_Location1/7(In7)
  Median_Accumulator1/8(Out8) -> Median_Location1/8(In8)
  Median_Location1/1(Out1) -> Scope1/1, Mux2/3
  med_loc/1 -> Median_Location1/9(median_loc), Median_Location2/9(median_loc)
  Median_Accumulator2/8(Out8) -> Median_Location2/8(In8)
  Median_Accumulator2/7(Out7) -> Median_Location2/7(In7)
  Median_Accumulator2/6(Out6) -> Median_Location2/6(In6)
  Median_Accumulator2/5(Out5) -> Median_Location2/5(In5)
  Median_Accumulator2/4(Out4) -> Median_Location2/4(In4)
  Median_Accumulator2/3(Out3) -> Median_Location2/3(In3)
  Median_Accumulator2/2(Out2) -> Median_Location2/2(In2)
  Median_Accumulator2/1(Out1) -> Median_Location2/1(In1)
  Constant9/1 -> Mux/2
  Constant10/1 -> Mux/3
  Mux/1 -> Logical1/1, Mux2/1
  Inverter/1 -> Logical/1
  Logical/1 -> Mux1/1
  Constant11/1 -> Mux1/2
  Mux1/1 -> Logical1/2, Mux3/1
  Median_Location2/1(Out3) -> Mux3/3, Scope2/1, Scope4/2
  Constant12/1 -> Mux1/3
  Mux2/1 -> Logical2/1
  Mux3/1 -> Logical2/2
  Constant2/1 -> Mux2/2
  Constant3/1 -> Mux3/2
  Logical2/1 -> Scope3/2, med_calc1/1
  Logical1/1 -> priority_out1/1, Scope3/1
  Median_Accumulator2/9(inc_signal) -> inc_out/1
  Median_Location1/2(sel1) -> Mux/1, Inverter/1, Scope1/2
  Median_Location2/2(sel2) -> Logical/2, Scope2/2
  acc_reset/1 -> Median_Accumulator1/2(acc_reset), Median_Accumulator2/2(acc_reset)

== system rfi_blk_v6_casper/rfi_blk/mad_comp/Median1/Subsystem3 ==
  data_in : Inport  OutDataType=sfix(16)  OutScaling=2^0
  med_loc : Inport  Port=2  OutDataType=sfix(16)  OutScaling=2^0
  acc_reset : Inport  Port=3  OutDataType=sfix(16)  OutScaling=2^0
  " System Generator" : xbsIndex_r4/ System Generator  Tag=genX  SystemSampleTime=-1  xilinxfamily=Virtex4  part=xc4vsx35  speed=-10  package=ff668  synthesis_tool=XST  clock_wrapper=Clock Enables  directory=./netlist  testbench=off  simulink_period=1  sysclk_period=100  dcm_input_clock_period=100  incr_netlist=off  trim_vbits=Everywhere in SubSystem  dbl_ovrd=According to Block Masks  core_generation=According to Block Masks  run_coregen=off  deprecated_control=off  eval_field=0  block_type=sysgen
      settings: block_version=10.1.3
  Constant10 : xbsIndex_r4/Constant  arith_type=Boolean  const=1  n_bits=1  bin_pt=0  explicit_period=on  period=1  equ=P=C  opselect=C  inp2=PCIN>>17  opr=+  inp1=P  carry=CIN  dbl_ovrd=off  block_type=constant
  Constant11 : xbsIndex_r4/Constant  arith_type=Boolean  const=0  n_bits=1  bin_pt=0  explicit_period=on  period=1  equ=P=C  opselect=C  inp2=PCIN>>17  opr=+  inp1=P  carry=CIN  dbl_ovrd=off  block_type=constant
  Constant12 : xbsIndex_r4/Constant  arith_type=Boolean  const=1  n_bits=1  bin_pt=0  explicit_period=on  period=1  equ=P=C  opselect=C  inp2=PCIN>>17  opr=+  inp1=P  carry=CIN  dbl_ovrd=off  block_type=constant
  Constant2 : xbsIndex_r4/Constant  arith_type=Unsigned  const=0  n_bits=8  bin_pt=0  explicit_period=on  period=1  equ=P=C  opselect=C  inp2=PCIN>>17  opr=+  inp1=P  carry=CIN  dbl_ovrd=off  block_type=constant
  Constant3 : xbsIndex_r4/Constant  arith_type=Unsigned  const=0  n_bits=8  bin_pt=0  explicit_period=on  period=1  equ=P=C  opselect=C  inp2=PCIN>>17  opr=+  inp1=P  carry=CIN  dbl_ovrd=off  block_type=constant
  Constant9 : xbsIndex_r4/Constant  arith_type=Boolean  const=0  n_bits=1  bin_pt=0  explicit_period=on  period=1  equ=P=C  opselect=C  inp2=PCIN>>17  opr=+  inp1=P  carry=CIN  dbl_ovrd=off  block_type=constant
  Inverter : xbsIndex_r4/Inverter  en=off  latency=0  dbl_ovrd=off  block_type=inv
  Logical : xbsIndex_r4/Logical  logical_function=AND  inputs=2  en=off  latency=0  precision=Full  arith_type=Unsigned  n_bits=16  bin_pt=0  align_bp=on  dbl_ovrd=off  block_type=logical
  Logical1 : xbsIndex_r4/Logical  logical_function=OR  inputs=2  en=off  latency=1  precision=User Defined  arith_type=Unsigned  n_bits=1  bin_pt=0  align_bp=on  dbl_ovrd=off  block_type=logical
  Logical2 : xbsIndex_r4/Logical  logical_function=OR  inputs=2  en=off  latency=1  precision=User Defined  arith_type="Signed  (2's comp)"  n_bits=8  bin_pt=0  align_bp=on  dbl_ovrd=off  block_type=logical
  Median_Accumulator1 : Subsystem
  Median_Accumulator2 : Subsystem
  Median_Location1 : Subsystem
  Median_Location2 : Subsystem
  Mux : xbsIndex_r4/Mux  inputs=2  en=off  latency=0  precision=Full  arith_type=Unsigned  n_bits=16  bin_pt=14  quantization=Truncate  overflow=Wrap  dbl_ovrd=off  block_type=mux
  Mux1 : xbsIndex_r4/Mux  inputs=2  en=off  latency=0  precision=Full  arith_type="Signed  (2's comp)"  n_bits=8  bin_pt=0  quantization=Truncate  overflow=Wrap  dbl_ovrd=off  block_type=mux
  Mux2 : xbsIndex_r4/Mux  inputs=2  en=off  latency=0  precision=User Defined  arith_type="Signed  (2's comp)"  n_bits=8  bin_pt=0  quantization=Truncate  overflow=Wrap  dbl_ovrd=off  block_type=mux
  Mux3 : xbsIndex_r4/Mux  inputs=2  en=off  latency=0  precision=User Defined  arith_type="Signed  (2's comp)"  n_bits=8  bin_pt=0  quantization=Truncate  overflow=Wrap  dbl_ovrd=off  block_type=mux
  Scope{1..5} (5 alike) : Scope  NumInputPorts=2
      Scope1 settings: YMin=-5~-5  YMax=5~5
      Scope2 settings: YMin=-5~-5  YMax=5~5
      Scope3 settings: YMin=-5~-5  YMax=5~5  SaveName=ScopeData5
      Scope4 settings: YMin=-5~-5  YMax=5~5  SaveName=ScopeData7
      Scope5 settings: YMin=-5~-5  YMax=5~5  SaveName=ScopeData15
  priority_out1 : Outport  OutDataType=sfix(16)  OutScaling=2^0
  med_calc1 : Outport  Port=2  OutDataType=sfix(16)  OutScaling=2^0
  inc_out : Outport  Port=3
  wires:
  data_in/1 -> Median_Accumulator1/1(data_in), Median_Accumulator2/1(data_in)
  Median_Accumulator1/1(Out1) -> Median_Location1/1(In1), Scope5/1
  Median_Accumulator1/2(Out2) -> Median_Location1/2(In2)
  Median_Accumulator1/3(Out3) -> Median_Location1/3(In3)
  Median_Accumulator1/4(Out4) -> Median_Location1/4(In4)
  Median_Accumulator1/5(Out5) -> Median_Location1/5(In5), Scope5/2
  Median_Accumulator1/6(Out6) -> Median_Location1/6(In6)
  Median_Accumulator1/7(Out7) -> Median_Location1/7(In7)
  Median_Accumulator1/8(Out8) -> Median_Location1/8(In8)
  Median_Location1/1(Out1) -> Scope1/1, Mux2/3
  med_loc/1 -> Median_Location1/9(median_loc), Median_Location2/9(median_loc)
  Median_Accumulator2/8(Out8) -> Median_Location2/8(In8)
  Median_Accumulator2/7(Out7) -> Median_Location2/7(In7)
  Median_Accumulator2/6(Out6) -> Median_Location2/6(In6)
  Median_Accumulator2/5(Out5) -> Median_Location2/5(In5)
  Median_Accumulator2/4(Out4) -> Median_Location2/4(In4)
  Median_Accumulator2/3(Out3) -> Median_Location2/3(In3)
  Median_Accumulator2/2(Out2) -> Median_Location2/2(In2)
  Median_Accumulator2/1(Out1) -> Median_Location2/1(In1)
  Constant9/1 -> Mux/2
  Constant10/1 -> Mux/3
  Mux/1 -> Logical1/1, Mux2/1
  Inverter/1 -> Logical/1
  Logical/1 -> Mux1/1
  Constant11/1 -> Mux1/2
  Mux1/1 -> Logical1/2, Mux3/1
  Median_Location2/1(Out3) -> Mux3/3, Scope2/1, Scope4/2
  Constant12/1 -> Mux1/3
  Mux2/1 -> Logical2/1
  Mux3/1 -> Logical2/2
  Constant2/1 -> Mux2/2
  Constant3/1 -> Mux3/2
  Logical2/1 -> Scope3/2, med_calc1/1
  Logical1/1 -> priority_out1/1, Scope3/1
  Median_Accumulator2/9(inc_signal) -> inc_out/1
  Median_Location1/2(sel1) -> Mux/1, Inverter/1, Scope1/2
  Median_Location2/2(sel2) -> Logical/2, Scope2/2
  acc_reset/1 -> Median_Accumulator1/2(acc_reset), Median_Accumulator2/2(acc_reset)
